simulink model "AcasXuClosedLoop_all"  (Simulink 10.0)
settings: Solver=VariableStepAuto  StartTime=0.0  StopTime=20  RelTol=1e-3
libraries: neural x14040, simulink x2
(part 36 of 534)

== system AcasXuClosedLoop_all/networks/Networks_m_9/AcasXu_1_9/Layer 3 ==
  "a{2} " : Inport  PortDimensions=50
      mask Display: e = 0.06;c1 = [120 230 180]/255;c2 = ([120 230 180]+0)/(256+128);c3 = ([120 230 180]+128)/(256+128);patch([0 1 1 0],[0 0 1 1],c1);patch([0 1 1 1-e 1-e 0+e],[0 0 1 1-e 0+e 0+e],c2);patch([0 0 1 1-e 0+e 0+e],[0 1 1 1-e 1-e 0+e],c3);plot([0 1 1 0 0],[0 0 1 1 0]);
  Delays 1 : Subsystem
      mask Display: e = 0.06;c1 = [120 150 230]/255;c2 = ([120 150 230]+0)/383;c3 = ([120 150 230]+128)/383;patch([0 1 1 0],[0 0 1 1],c1);patch([0 1 1 1-e 1-e 0+e],[0 0 1 1-e 0+e 0+e],c2);patch([0 0 1 1-e 0+e 0+e],[0 1 1 1-e 1-e 0+e],c3);plot([0 1 1 0 0],[0 0 1 1 0]);disp('0');
  "LW{3,2}" : Subsystem
      mask Display: e = 0.06;c1 = [120 150 230]/255;c2 = ([120 150 230]+0)/383;c3 = ([120 150 230]+128)/383;patch([0 1 1 0],[0 0 1 1],c1);patch([0 1 1 1-e 1-e 0+e],[0 0 1 1-e 0+e 0+e],c2);patch([0 0 1 1-e 0+e 0+e],[0 1 1 1-e 1-e 0+e],c3);plot([0 1 1 0 0],[0 0 1 1 0]);disp('W');
  "b{3}" : Constant  Value=[0.579304000000000041126213545794598758220672607421875;0.1281029999999999946513895565658458508551120758056640625;-0.1748010000000000119246834628938813693821430206298828125;-0.0029703199999999998180688631777002228773199021816253662109375;-0.057232600000000001527755699726185412146151065826416015625;-0.257135999999999975695885723325773142278194427490234375;-0.0175342999999999991922461362037211074493825435638427734375;0.2345440000000000024815705046421498991549015045166015625;-0.000781700999999999988736287814816705576959066092967987060546875;-0.00547454999999999990245580505643374635837972164154052734375;-0.04520269999999999843698361701171961612999439239501953125;-0.2583699999999999885602619542623870074748992919921875;-0.0831357000000000068151706500430009327828884124755859375;0.06992959999999999454178833957485039718449115753173828125;-0.04588620000000000198614458213342004455626010894775390625;-0.162262999999999990574650610142271034419536590576171875;0.000353693999999999991908083973868315297295339405536651611328125;0.2176039999999999918767201734226546250283718109130859375;0.1180549999999999932764893628700519911944866180419921875;0.044857800000000003226574563086614944040775299072265625;-0.6526009999999999866560074224253185093402862548828125;0.4370629999999999792947846799506805837154388427734375;0.06949550000000000171684888528034207411110401153564453125;-0.05631350000000000244337883259504451416432857513427734375;-0.2005689999999999972857267493964172899723052978515625;-0.61900800000000000267164068645797669887542724609375;0.07579249999999999876099110451832530088722705841064453125;0.164617999999999986560084153097704984247684478759765625;-0.6353269999999999750883716842508874833583831787109375;0.0186143000000000002902567075580009259283542633056640625;0.18131099999999999994315658113919198513031005859375;0.047283499999999999141575557359828962944447994232177734375;-0.340189000000000019152679442413500510156154632568359375;0.43907200000000001782751724022091366350650787353515625;0.263168000000000013027801060161436907947063446044921875;0.017979900000000000048760995241536875255405902862548828125;-0.00601458000000000027329694063382703461684286594390869140625;0.08392919999999999547402040889210184104740619659423828125;0.59921100000000004914824103252612985670566558837890625;0.10220300000000000217870166352440719492733478546142578125;0.303765000000000007229772336359019391238689422607421875;0.163652999999999992919441638150601647794246673583984375;-0.029870500000000001217248524199021630920469760894775390625;0.1906400000000000038991032624835497699677944183349609375;0.01548440000000000059399152263495125225745141506195068359375;-0.2505100000000000104449782156734727323055267333984375;-0.1780980000000000063042904230314888991415500640869140625;-0.2264130000000000031423752488990430720150470733642578125;-0.048898299999999998710631388121328200213611125946044921875;-0.12159599999999999575539533225310151465237140655517578125]
      mask Display: e = 0.06;c1 = [120 150 230]/255;c2 = ([120 150 230]+0)/383;c3 = ([120 150 230]+128)/383;patch([0 1 1 0],[0 0 1 1],c1);patch([0 1 1 1-e 1-e 0+e],[0 0 1 1-e 0+e 0+e],c2);patch([0 0 1 1-e 0+e 0+e],[0 1 1 1-e 1-e 0+e],c3);plot([0 1 1 0 0],[0 0 1 1 0]);disp('b');
  netsum : Sum  Inputs=2  SaturateOnIntegerOverflow=on  InputSameDT=on  OutDataTypeStr=Inherit: Same as first input
      mask Display: e = 0.06;c1 = [120 150 230]/255;c2 = ([120 150 230]+0)/383;c3 = ([120 150 230]+128)/383;patch([0 1 1 0],[0 0 1 1],c1);patch([0 1 1 1-e 1-e 0+e],[0 0 1 1-e 0+e 0+e],c2);patch([0 0 1 1-e 0+e 0+e],[0 1 1 1-e 1-e 0+e],c3);plot([0 1 1 0 0],[0 0 1 1 0]);disp('+');
  poslin : neural/Transfer Functions/poslin
      settings: SourceProductName=Deep Learning Toolbox  SourceProductBaseCode=NN
  "a{3}" : Outport  InitialOutput=[0;0;0;0;0;0;0;0;0;0;0;0;0;0;0;0;0;0;0;0;0;0;0;0;0;0;0;0;0;0;0;0;0;0;0;0;0;0;0;0;0;0;0;0;0;0;0;0;0;0]
      mask Display: e = 0.06;c1 = [120 230 180]/255;c2 = ([120 230 180]+0)/(256+128);c3 = ([120 230 180]+128)/(256+128);patch([0 1 1 0],[0 0 1 1],c1);patch([0 1 1 1-e 1-e 0+e],[0 0 1 1-e 0+e 0+e],c2);patch([0 0 1 1-e 0+e 0+e],[0 1 1 1-e 1-e 0+e],c3);plot([0 1 1 0 0],[0 0 1 1 0]);
  wires:
  poslin/1 -> "a{3}"/1
  netsum/1 -> poslin/1
  "b{3}"/1 -> netsum/2
  "LW{3,2}"/1("lz{3,2}") -> netsum/1
  Delays 1/1("pd{3,2}") -> "LW{3,2}"/1("ad{3,2}")
  "a{2} "/1 -> Delays 1/1("p{3}")

== system AcasXuClosedLoop_all/networks/Networks_m_9/AcasXu_1_9/Layer 4 ==
  "a{3} " : Inport  PortDimensions=50
      mask Display: e = 0.06;c1 = [120 230 180]/255;c2 = ([120 230 180]+0)/(256+128);c3 = ([120 230 180]+128)/(256+128);patch([0 1 1 0],[0 0 1 1],c1);patch([0 1 1 1-e 1-e 0+e],[0 0 1 1-e 0+e 0+e],c2);patch([0 0 1 1-e 0+e 0+e],[0 1 1 1-e 1-e 0+e],c3);plot([0 1 1 0 0],[0 0 1 1 0]);
  Delays 1 : Subsystem
      mask Display: e = 0.06;c1 = [120 150 230]/255;c2 = ([120 150 230]+0)/383;c3 = ([120 150 230]+128)/383;patch([0 1 1 0],[0 0 1 1],c1);patch([0 1 1 1-e 1-e 0+e],[0 0 1 1-e 0+e 0+e],c2);patch([0 0 1 1-e 0+e 0+e],[0 1 1 1-e 1-e 0+e],c3);plot([0 1 1 0 0],[0 0 1 1 0]);disp('0');
  "LW{4,3}" : Subsystem
      mask Display: e = 0.06;c1 = [120 150 230]/255;c2 = ([120 150 230]+0)/383;c3 = ([120 150 230]+128)/383;patch([0 1 1 0],[0 0 1 1],c1);patch([0 1 1 1-e 1-e 0+e],[0 0 1 1-e 0+e 0+e],c2);patch([0 0 1 1-e 0+e 0+e],[0 1 1 1-e 1-e 0+e],c3);plot([0 1 1 0 0],[0 0 1 1 0]);disp('W');
  "b{4}" : Constant  Value=[0.00952038000000000016187495788244632421992719173431396484375;-0.343938000000000021483259615706629119813442230224609375;1.177820000000000089102059064316563308238983154296875;-0.228142000000000011450396186774014495313167572021484375;-0.294987999999999972455810848259716294705867767333984375;-0.03578710000000000224229523837493616156280040740966796875;-0.257523000000000001907807245515868999063968658447265625;-0.255493000000000025639934619903215207159519195556640625;-0.280859000000000025298874106738367117941379547119140625;-0.2443720000000000058815174952542292885482311248779296875;-0.1862540000000000028901325777042075060307979583740234375;-0.08710220000000000462048177496399148367345333099365234375;-0.050596099999999998020339120330390869639813899993896484375;-0.04549129999999999840465392253463505767285823822021484375;-0.1145899999999999974154007986726355738937854766845703125;-0.1090150000000000007904787935331114567816257476806640625;-0.81820400000000004236966333337477408349514007568359375;-0.252141000000000004011013743365765549242496490478515625;0.43712099999999998178878968246863223612308502197265625;-0.0166662999999999984768184191352702328003942966461181640625;-0.317574000000000022936319510336033999919891357421875;-0.06494189999999999685087459511123597621917724609375;-0.8847620000000000484163820146932266652584075927734375;-0.02530469999999999941575623552125762216746807098388671875;0.07968530000000000057536198028174112550914287567138671875;-0.2003979999999999928039784435895853675901889801025390625;0.320222999999999979880982436952763237059116363525390625;-0.333703000000000027380764322515460662543773651123046875;0.1671620000000000050288662123421090655028820037841796875;-0.014145100000000000728395121996072703041136264801025390625;-0.37539800000000000945732381296693347394466400146484375;-0.90470600000000001017497197608463466167449951171875;0.05014369999999999938378181241205311380326747894287109375;-0.257811999999999985622167741894372738897800445556640625;-0.00607064000000000041079584178760342183522880077362060546875;0.34403600000000000846966941026039421558380126953125;-0.0242753999999999990622168155596227734349668025970458984375;-0.295009000000000021213253376117791049182415008544921875;-0.1626789999999999902779990179624292068183422088623046875;-0.325608999999999981778131541432230733335018157958984375;0.4586040000000000116386900117504410445690155029296875;0.15100700000000000233768560065072961151599884033203125;-0.01311299999999999965627495157605153508484363555908203125;-0.380357000000000000650146603220491670072078704833984375;0.0221107000000000004924505248027344350703060626983642578125;-0.10982599999999999307309650475872331298887729644775390625;-0.1503339999999999954116702838291530497372150421142578125;-0.01328509999999999934006122970231444924138486385345458984375;-0.04880589999999999928359528666987898759543895721435546875;-0.046639199999999998713651194748308626003563404083251953125]
      mask Display: e = 0.06;c1 = [120 150 230]/255;c2 = ([120 150 230]+0)/383;c3 = ([120 150 230]+128)/383;patch([0 1 1 0],[0 0 1 1],c1);patch([0 1 1 1-e 1-e 0+e],[0 0 1 1-e 0+e 0+e],c2);patch([0 0 1 1-e 0+e 0+e],[0 1 1 1-e 1-e 0+e],c3);plot([0 1 1 0 0],[0 0 1 1 0]);disp('b');
  netsum : Sum  Inputs=2  SaturateOnIntegerOverflow=on  InputSameDT=on  OutDataTypeStr=Inherit: Same as first input
      mask Display: e = 0.06;c1 = [120 150 230]/255;c2 = ([120 150 230]+0)/383;c3 = ([120 150 230]+128)/383;patch([0 1 1 0],[0 0 1 1],c1);patch([0 1 1 1-e 1-e 0+e],[0 0 1 1-e 0+e 0+e],c2);patch([0 0 1 1-e 0+e 0+e],[0 1 1 1-e 1-e 0+e],c3);plot([0 1 1 0 0],[0 0 1 1 0]);disp('+');
  poslin : neural/Transfer Functions/poslin
      settings: SourceProductName=Deep Learning Toolbox  SourceProductBaseCode=NN
  "a{4}" : Outport  InitialOutput=[0;0;0;0;0;0;0;0;0;0;0;0;0;0;0;0;0;0;0;0;0;0;0;0;0;0;0;0;0;0;0;0;0;0;0;0;0;0;0;0;0;0;0;0;0;0;0;0;0;0]
      mask Display: e = 0.06;c1 = [120 230 180]/255;c2 = ([120 230 180]+0)/(256+128);c3 = ([120 230 180]+128)/(256+128);patch([0 1 1 0],[0 0 1 1],c1);patch([0 1 1 1-e 1-e 0+e],[0 0 1 1-e 0+e 0+e],c2);patch([0 0 1 1-e 0+e 0+e],[0 1 1 1-e 1-e 0+e],c3);plot([0 1 1 0 0],[0 0 1 1 0]);
  wires:
  poslin/1 -> "a{4}"/1
  netsum/1 -> poslin/1
  "b{4}"/1 -> netsum/2
  "LW{4,3}"/1("lz{4,3}") -> netsum/1
  Delays 1/1("pd{4,3}") -> "LW{4,3}"/1("ad{4,3}")
  "a{3} "/1 -> Delays 1/1("p{4}")

== system AcasXuClosedLoop_all/networks/Networks_m_9/AcasXu_1_9/Layer 5 ==
  "a{4} " : Inport  PortDimensions=50
      mask Display: e = 0.06;c1 = [120 230 180]/255;c2 = ([120 230 180]+0)/(256+128);c3 = ([120 230 180]+128)/(256+128);patch([0 1 1 0],[0 0 1 1],c1);patch([0 1 1 1-e 1-e 0+e],[0 0 1 1-e 0+e 0+e],c2);patch([0 0 1 1-e 0+e 0+e],[0 1 1 1-e 1-e 0+e],c3);plot([0 1 1 0 0],[0 0 1 1 0]);
  Delays 1 : Subsystem
      mask Display: e = 0.06;c1 = [120 150 230]/255;c2 = ([120 150 230]+0)/383;c3 = ([120 150 230]+128)/383;patch([0 1 1 0],[0 0 1 1],c1);patch([0 1 1 1-e 1-e 0+e],[0 0 1 1-e 0+e 0+e],c2);patch([0 0 1 1-e 0+e 0+e],[0 1 1 1-e 1-e 0+e],c3);plot([0 1 1 0 0],[0 0 1 1 0]);disp('0');
  "LW{5,4}" : Subsystem
      mask Display: e = 0.06;c1 = [120 150 230]/255;c2 = ([120 150 230]+0)/383;c3 = ([120 150 230]+128)/383;patch([0 1 1 0],[0 0 1 1],c1);patch([0 1 1 1-e 1-e 0+e],[0 0 1 1-e 0+e 0+e],c2);patch([0 0 1 1-e 0+e 0+e],[0 1 1 1-e 1-e 0+e],c3);plot([0 1 1 0 0],[0 0 1 1 0]);disp('W');
  "b{5}" : Constant  Value=[-0.29072399999999998243538357201032340526580810546875;0.0272885999999999996623589737509973929263651371002197265625;0.1793730000000000046611603465862572193145751953125;-0.07173649999999999471445022436455474235117435455322265625;-0.234843999999999997196908907426404766738414764404296875;-0.85785699999999998066613215996767394244670867919921875;-0.00822291999999999988213428281369488104246556758880615234375;0.265643000000000018001600210482138209044933319091796875;-1.3297399999999999220534618871170096099376678466796875;0.1350659999999999916209247885490185581147670745849609375;-0.148589999999999999857891452847979962825775146484375;0.08024480000000000501358954352326691150665283203125;0.26909199999999999786126636536209844052791595458984375;-0.285260000000000013553602684623911045491695404052734375;0.1955969999999999930917482515724259428679943084716796875;0.3249020000000000241158204516978003084659576416015625;0.494925000000000003819167204710538499057292938232421875;-0.06360369999999999912621007069901679642498493194580078125;-0.014471299999999999441602227534531266428530216217041015625;-0.302879999999999982573939405483542941510677337646484375;-0.1371610000000000051390003363849245943129062652587890625;-1.131040000000000045332626541494391858577728271484375;-0.440622999999999986897591952583752572536468505859375;0.03643760000000000054409809990829671733081340789794921875;0.1914639999999999953050888734651380218565464019775390625;0.17777399999999998758681840627104975283145904541015625;-0.449446999999999985409004921166342683136463165283203125;-0.1299329999999999929460869907416054047644138336181640625;-0.018397299999999998376676302314081112854182720184326171875;-0.019312400000000000399591471023086342029273509979248046875;0.10747600000000000208810746471499442122876644134521484375;0.1691249999999999975575093458246556110680103302001953125;-0.044454500000000000958788604066285188309848308563232421875;0.250553999999999998937738610038650222122669219970703125;-0.331932999999999978069098460764507763087749481201171875;0.1414890000000000036539660186463152058422565460205078125;0.1969249999999999889421786747334408573806285858154296875;-0.768086999999999964217067827121354639530181884765625;0.06017229999999999801030270418777945451438426971435546875;-1.236050000000000093081098384573124349117279052734375;-0.93613400000000002165734258596785366535186767578125;-0.1641060000000000018705037518884637393057346343994140625;-0.217586000000000001630695578569429926574230194091796875;-0.0254946999999999986130649887172694434411823749542236328125;-0.174792000000000002923883357652812264859676361083984375;-0.1638570000000000026485480475457734428346157073974609375;-2.14449999999999985078602549037896096706390380859375;-0.459907000000000010242473535981844179332256317138671875;-0.09570120000000000015649703755116206593811511993408203125;0.132589000000000012402523452692548744380474090576171875]
      mask Display: e = 0.06;c1 = [120 150 230]/255;c2 = ([120 150 230]+0)/383;c3 = ([120 150 230]+128)/383;patch([0 1 1 0],[0 0 1 1],c1);patch([0 1 1 1-e 1-e 0+e],[0 0 1 1-e 0+e 0+e],c2);patch([0 0 1 1-e 0+e 0+e],[0 1 1 1-e 1-e 0+e],c3);plot([0 1 1 0 0],[0 0 1 1 0]);disp('b');
  netsum : Sum  Inputs=2  SaturateOnIntegerOverflow=on  InputSameDT=on  OutDataTypeStr=Inherit: Same as first input
      mask Display: e = 0.06;c1 = [120 150 230]/255;c2 = ([120 150 230]+0)/383;c3 = ([120 150 230]+128)/383;patch([0 1 1 0],[0 0 1 1],c1);patch([0 1 1 1-e 1-e 0+e],[0 0 1 1-e 0+e 0+e],c2);patch([0 0 1 1-e 0+e 0+e],[0 1 1 1-e 1-e 0+e],c3);plot([0 1 1 0 0],[0 0 1 1 0]);disp('+');
  poslin : neural/Transfer Functions/poslin
      settings: SourceProductName=Deep Learning Toolbox  SourceProductBaseCode=NN
  "a{5}" : Outport  InitialOutput=[0;0;0;0;0;0;0;0;0;0;0;0;0;0;0;0;0;0;0;0;0;0;0;0;0;0;0;0;0;0;0;0;0;0;0;0;0;0;0;0;0;0;0;0;0;0;0;0;0;0]
      mask Display: e = 0.06;c1 = [120 230 180]/255;c2 = ([120 230 180]+0)/(256+128);c3 = ([120 230 180]+128)/(256+128);patch([0 1 1 0],[0 0 1 1],c1);patch([0 1 1 1-e 1-e 0+e],[0 0 1 1-e 0+e 0+e],c2);patch([0 0 1 1-e 0+e 0+e],[0 1 1 1-e 1-e 0+e],c3);plot([0 1 1 0 0],[0 0 1 1 0]);
  wires:
  poslin/1 -> "a{5}"/1
  netsum/1 -> poslin/1
  "b{5}"/1 -> netsum/2
  "LW{5,4}"/1("lz{5,4}") -> netsum/1
  Delays 1/1("pd{5,4}") -> "LW{5,4}"/1("ad{5,4}")
  "a{4} "/1 -> Delays 1/1("p{5}")

== system AcasXuClosedLoop_all/networks/Networks_m_9/AcasXu_1_9/Layer 6 ==
  "a{5} " : Inport  PortDimensions=50
      mask Display: e = 0.06;c1 = [120 230 180]/255;c2 = ([120 230 180]+0)/(256+128);c3 = ([120 230 180]+128)/(256+128);patch([0 1 1 0],[0 0 1 1],c1);patch([0 1 1 1-e 1-e 0+e],[0 0 1 1-e 0+e 0+e],c2);patch([0 0 1 1-e 0+e 0+e],[0 1 1 1-e 1-e 0+e],c3);plot([0 1 1 0 0],[0 0 1 1 0]);
  Delays 1 : Subsystem
      mask Display: e = 0.06;c1 = [120 150 230]/255;c2 = ([120 150 230]+0)/383;c3 = ([120 150 230]+128)/383;patch([0 1 1 0],[0 0 1 1],c1);patch([0 1 1 1-e 1-e 0+e],[0 0 1 1-e 0+e 0+e],c2);patch([0 0 1 1-e 0+e 0+e],[0 1 1 1-e 1-e 0+e],c3);plot([0 1 1 0 0],[0 0 1 1 0]);disp('0');
  "LW{6,5}" : Subsystem
      mask Display: e = 0.06;c1 = [120 150 230]/255;c2 = ([120 150 230]+0)/383;c3 = ([120 150 230]+128)/383;patch([0 1 1 0],[0 0 1 1],c1);patch([0 1 1 1-e 1-e 0+e],[0 0 1 1-e 0+e 0+e],c2);patch([0 0 1 1-e 0+e 0+e],[0 1 1 1-e 1-e 0+e],c3);plot([0 1 1 0 0],[0 0 1 1 0]);disp('W');
  "b{6}" : Constant  Value=[0.054117800000000000626432239414498326368629932403564453125;-0.029863699999999999967759123364885454066097736358642578125;-0.3050450000000000105870867628254927694797515869140625;-0.04563230000000000063931082650015014223754405975341796875;-0.91212400000000004585132273859926499426364898681640625;-0.38360300000000002729194648054544813930988311767578125;-1.2402599999999999180744225668604485690593719482421875;0.03821739999999999859436883298258180730044841766357421875;-0.003371550000000000067712502271888297400437295436859130859375;0.7082969999999999544115780736319720745086669921875;-0.0424785000000000023678836669205338694155216217041015625;-0.463994999999999990780708003512700088322162628173828125;-0.048301900000000001778044378397680702619254589080810546875;-0.47921599999999997532285078705172054469585418701171875;0.07510529999999999983817389193063718266785144805908203125;-0.053192499999999996729282969454288831911981105804443359375;0.1580640000000000100488506404872168786823749542236328125;-0.06372360000000000523900922644315869547426700592041015625;0.007425969999999999619777479864524138974957168102264404296875;-0.237055999999999988947507745251641608774662017822265625;-0.7746969999999999689777041567140258848667144775390625;0.000659087000000000040877967677488413755781948566436767578125;-0.357331999999999982975396051187999546527862548828125;0.0168764999999999991409094235450538690201938152313232421875;-0.0990453000000000027824853532365523278713226318359375;0.09667389999999999317115140229361713863909244537353515625;-0.045709800000000001818367678652066388167440891265869140625;0.1134050000000000057998050806418177671730518341064453125;0.1596950000000000036148861681795096956193447113037109375;-0.122028999999999998582467242158600129187107086181640625;-0.29037400000000002098232698699575848877429962158203125;-0.0639891999999999960824226263866876251995563507080078125;-0.0760119999999999962358998573108692653477191925048828125;-0.07896699999999999552979801364926970563828945159912109375;-0.3772699999999999942446038403431884944438934326171875;-0.04114099999999999701660868822727934457361698150634765625;0.0158231999999999990713650532825340633280575275421142578125;-0.76031700000000002059863390968530438840389251708984375;-0.2510149999999999881339363128063268959522247314453125;0.0304802999999999983671283843023047666065394878387451171875;-0.09740379999999999871551636942967888899147510528564453125;-0.03860149999999999692601448941786657087504863739013671875;0.05038600000000000023181456754173268564045429229736328125;0.05410210000000000019060308886764687485992908477783203125;-0.035352899999999999602895428552074008621275424957275390625;-0.268838999999999994638955058690044097602367401123046875;-0.04333900000000000252153853352865553461015224456787109375;-0.026029400000000001147437700410591787658631801605224609375;-0.1915530000000000010462741784067475236952304840087890625;0.041969499999999999861888255736630526371300220489501953125]
      mask Display: e = 0.06;c1 = [120 150 230]/255;c2 = ([120 150 230]+0)/383;c3 = ([120 150 230]+128)/383;patch([0 1 1 0],[0 0 1 1],c1);patch([0 1 1 1-e 1-e 0+e],[0 0 1 1-e 0+e 0+e],c2);patch([0 0 1 1-e 0+e 0+e],[0 1 1 1-e 1-e 0+e],c3);plot([0 1 1 0 0],[0 0 1 1 0]);disp('b');
  netsum : Sum  Inputs=2  SaturateOnIntegerOverflow=on  InputSameDT=on  OutDataTypeStr=Inherit: Same as first input
      mask Display: e = 0.06;c1 = [120 150 230]/255;c2 = ([120 150 230]+0)/383;c3 = ([120 150 230]+128)/383;patch([0 1 1 0],[0 0 1 1],c1);patch([0 1 1 1-e 1-e 0+e],[0 0 1 1-e 0+e 0+e],c2);patch([0 0 1 1-e 0+e 0+e],[0 1 1 1-e 1-e 0+e],c3);plot([0 1 1 0 0],[0 0 1 1 0]);disp('+');
  poslin : neural/Transfer Functions/poslin
      settings: SourceProductName=Deep Learning Toolbox  SourceProductBaseCode=NN
  "a{6}" : Outport  InitialOutput=[0;0;0;0;0;0;0;0;0;0;0;0;0;0;0;0;0;0;0;0;0;0;0;0;0;0;0;0;0;0;0;0;0;0;0;0;0;0;0;0;0;0;0;0;0;0;0;0;0;0]
      mask Display: e = 0.06;c1 = [120 230 180]/255;c2 = ([120 230 180]+0)/(256+128);c3 = ([120 230 180]+128)/(256+128);patch([0 1 1 0],[0 0 1 1],c1);patch([0 1 1 1-e 1-e 0+e],[0 0 1 1-e 0+e 0+e],c2);patch([0 0 1 1-e 0+e 0+e],[0 1 1 1-e 1-e 0+e],c3);plot([0 1 1 0 0],[0 0 1 1 0]);
  wires:
  poslin/1 -> "a{6}"/1
  netsum/1 -> poslin/1
  "b{6}"/1 -> netsum/2
  "LW{6,5}"/1("lz{6,5}") -> netsum/1
  Delays 1/1("pd{6,5}") -> "LW{6,5}"/1("ad{6,5}")
  "a{5} "/1 -> Delays 1/1("p{6}")

== system AcasXuClosedLoop_all/networks/Networks_m_9/AcasXu_1_9/Layer 7 ==
  "a{6} " : Inport  PortDimensions=50
      mask Display: e = 0.06;c1 = [120 230 180]/255;c2 = ([120 230 180]+0)/(256+128);c3 = ([120 230 180]+128)/(256+128);patch([0 1 1 0],[0 0 1 1],c1);patch([0 1 1 1-e 1-e 0+e],[0 0 1 1-e 0+e 0+e],c2);patch([0 0 1 1-e 0+e 0+e],[0 1 1 1-e 1-e 0+e],c3);plot([0 1 1 0 0],[0 0 1 1 0]);
  Delays 1 : Subsystem
      mask Display: e = 0.06;c1 = [120 150 230]/255;c2 = ([120 150 230]+0)/383;c3 = ([120 150 230]+128)/383;patch([0 1 1 0],[0 0 1 1],c1);patch([0 1 1 1-e 1-e 0+e],[0 0 1 1-e 0+e 0+e],c2);patch([0 0 1 1-e 0+e 0+e],[0 1 1 1-e 1-e 0+e],c3);plot([0 1 1 0 0],[0 0 1 1 0]);disp('0');
  "LW{7,6}" : Subsystem
      mask Display: e = 0.06;c1 = [120 150 230]/255;c2 = ([120 150 230]+0)/383;c3 = ([120 150 230]+128)/383;patch([0 1 1 0],[0 0 1 1],c1);patch([0 1 1 1-e 1-e 0+e],[0 0 1 1-e 0+e 0+e],c2);patch([0 0 1 1-e 0+e 0+e],[0 1 1 1-e 1-e 0+e],c3);plot([0 1 1 0 0],[0 0 1 1 0]);disp('W');
  "b{7}" : Constant  Value=[-0.0201197000000000007779998867363246972672641277313232421875;-0.019449399999999998633892772659237380139529705047607421875;-0.0194817000000000010995204746677700313739478588104248046875;-0.0195344999999999997808419749389940989203751087188720703125;-0.01952500000000000068833827526759705506265163421630859375]
      mask Display: e = 0.06;c1 = [120 150 230]/255;c2 = ([120 150 230]+0)/383;c3 = ([120 150 230]+128)/383;patch([0 1 1 0],[0 0 1 1],c1);patch([0 1 1 1-e 1-e 0+e],[0 0 1 1-e 0+e 0+e],c2);patch([0 0 1 1-e 0+e 0+e],[0 1 1 1-e 1-e 0+e],c3);plot([0 1 1 0 0],[0 0 1 1 0]);disp('b');
  netsum : Sum  Inputs=2  SaturateOnIntegerOverflow=on  InputSameDT=on  OutDataTypeStr=Inherit: Same as first input
      mask Display: e = 0.06;c1 = [120 150 230]/255;c2 = ([120 150 230]+0)/383;c3 = ([120 150 230]+128)/383;patch([0 1 1 0],[0 0 1 1],c1);patch([0 1 1 1-e 1-e 0+e],[0 0 1 1-e 0+e 0+e],c2);patch([0 0 1 1-e 0+e 0+e],[0 1 1 1-e 1-e 0+e],c3);plot([0 1 1 0 0],[0 0 1 1 0]);disp('+');
  purelin : neural/Transfer Functions/purelin
      settings: SourceProductName=Deep Learning Toolbox
  "a{7}" : Outport  InitialOutput=[0;0;0;0;0]
      mask Display: e = 0.06;c1 = [120 230 180]/255;c2 = ([120 230 180]+0)/(256+128);c3 = ([120 230 180]+128)/(256+128);patch([0 1 1 0],[0 0 1 1],c1);patch([0 1 1 1-e 1-e 0+e],[0 0 1 1-e 0+e 0+e],c2);patch([0 0 1 1-e 0+e 0+e],[0 1 1 1-e 1-e 0+e],c3);plot([0 1 1 0 0],[0 0 1 1 0]);
  wires:
  purelin/1 -> "a{7}"/1
  netsum/1 -> purelin/1
  "b{7}"/1 -> netsum/2
  "LW{7,6}"/1("lz{7,6}") -> netsum/1
  Delays 1/1("pd{7,6}") -> "LW{7,6}"/1("ad{7,6}")
  "a{6} "/1 -> Delays 1/1("p{7}")

== system AcasXuClosedLoop_all/networks/Networks_m_9/AcasXu_1_9/Process Input 1 ==
  x : Inport  PortDimensions=5
      mask Display: e = 0.06;c1 = [120 230 180]/255;c2 = ([120 230 180]+0)/(256+128);c3 = ([120 230 180]+128)/(256+128);patch([0 1 1 0],[0 0 1 1],c1);patch([0 1 1 1-e 1-e 0+e],[0 0 1 1-e 0+e 0+e],c2);patch([0 0 1 1-e 0+e 0+e],[0 1 1 1-e 1-e 0+e],c3);plot([0 1 1 0 0],[0 0 1 1 0]);
  p : Outport  PortDimensions=5
      mask Display: e = 0.06;c1 = [120 230 180]/255;c2 = ([120 230 180]+0)/(256+128);c3 = ([120 230 180]+128)/(256+128);patch([0 1 1 0],[0 0 1 1],c1);patch([0 1 1 1-e 1-e 0+e],[0 0 1 1-e 0+e 0+e],c2);patch([0 0 1 1-e 0+e 0+e],[0 1 1 1-e 1-e 0+e],c3);plot([0 1 1 0 0],[0 0 1 1 0]);
  wires:
  x/1 -> p/1

== system AcasXuClosedLoop_all/networks/Networks_m_9/AcasXu_1_9/Process Output 1 ==
  a : Inport  PortDimensions=5
      mask Display: e = 0.06;c1 = [120 230 180]/255;c2 = ([120 230 180]+0)/(256+128);c3 = ([120 230 180]+128)/(256+128);patch([0 1 1 0],[0 0 1 1],c1);patch([0 1 1 1-e 1-e 0+e],[0 0 1 1-e 0+e 0+e],c2);patch([0 0 1 1-e 0+e 0+e],[0 1 1 1-e 1-e 0+e],c3);plot([0 1 1 0 0],[0 0 1 1 0]);
  y : Outport  PortDimensions=5
      mask Display: e = 0.06;c1 = [120 230 180]/255;c2 = ([120 230 180]+0)/(256+128);c3 = ([120 230 180]+128)/(256+128);patch([0 1 1 0],[0 0 1 1],c1);patch([0 1 1 1-e 1-e 0+e],[0 0 1 1-e 0+e 0+e],c2);patch([0 0 1 1-e 0+e 0+e],[0 1 1 1-e 1-e 0+e],c3);plot([0 1 1 0 0],[0 0 1 1 0]);
  wires:
  a/1 -> y/1

== system AcasXuClosedLoop_all/networks/Networks_m_9/AcasXu_2_9/Layer 1 ==
  "p{1}" : Inport  PortDimensions=5
      mask Display: e = 0.06;c1 = [120 230 180]/255;c2 = ([120 230 180]+0)/(256+128);c3 = ([120 230 180]+128)/(256+128);patch([0 1 1 0],[0 0 1 1],c1);patch([0 1 1 1-e 1-e 0+e],[0 0 1 1-e 0+e 0+e],c2);patch([0 0 1 1-e 0+e 0+e],[0 1 1 1-e 1-e 0+e],c3);plot([0 1 1 0 0],[0 0 1 1 0]);
  Delays 1 : Subsystem
      mask Display: e = 0.06;c1 = [120 150 230]/255;c2 = ([120 150 230]+0)/383;c3 = ([120 150 230]+128)/383;patch([0 1 1 0],[0 0 1 1],c1);patch([0 1 1 1-e 1-e 0+e],[0 0 1 1-e 0+e 0+e],c2);patch([0 0 1 1-e 0+e 0+e],[0 1 1 1-e 1-e 0+e],c3);plot([0 1 1 0 0],[0 0 1 1 0]);disp('0');
  "IW{1,1}" : Subsystem
      mask Display: e = 0.06;c1 = [120 150 230]/255;c2 = ([120 150 230]+0)/383;c3 = ([120 150 230]+128)/383;patch([0 1 1 0],[0 0 1 1],c1);patch([0 1 1 1-e 1-e 0+e],[0 0 1 1-e 0+e 0+e],c2);patch([0 0 1 1-e 0+e 0+e],[0 1 1 1-e 1-e 0+e],c3);plot([0 1 1 0 0],[0 0 1 1 0]);disp('W');
  "b{1}" : Constant  Value=[0.3133690000000000086544105215580202639102935791015625;-0.2295379999999999920401450026474776677787303924560546875;-0.68179599999999995763033666662522591650485992431640625;0.093745899999999993124077946049510501325130462646484375;-0.370910999999999990706101016257889568805694580078125;-0.08987399999999999555821972307967371307313442230224609375;0.241037000000000001254107928616576828062534332275390625;-0.0899728999999999945469397744091111235320568084716796875;0.01202310000000000010655920590352252474986016750335693359375;0.083605600000000002136602006430621258914470672607421875;-0.071525000000000005240252676230738870799541473388671875;0.1094549999999999967403851997005403973162174224853515625;0.211033999999999999364064251494710333645343780517578125;-0.201085000000000013731238368563936091959476470947265625;0.037052899999999999725019961260841228067874908447265625;-0.283455000000000012505552149377763271331787109375;0.1366680000000000116955334306112490594387054443359375;-0.63761199999999995657873341770027764141559600830078125;0.09052100000000000423749924038929748348891735076904296875;-0.017260299999999999254196580977804842405021190643310546875;-0.31535699999999999842970055397017858922481536865234375;0.08521869999999999445439158307635807432234287261962890625;-0.478976000000000012857270803579012863337993621826171875;-0.40773599999999998733102302139741368591785430908203125;-0.1191939999999999943991468853710102848708629608154296875;0.11499099999999999599520350557213532738387584686279296875;-0.463243000000000015869972003201837651431560516357421875;-0.348663000000000000699884594723698683083057403564453125;-0.351308000000000009155343150268890894949436187744140625;-0.26433600000000001539746108392137102782726287841796875;-0.2139709999999999945341500051654293201863765716552734375;-0.0735065000000000023927526626721373759210109710693359375;0.1385329999999999894821911539111169986426830291748046875;-0.497441999999999995285548948231735266745090484619140625;0.14723900000000000876099193192203529179096221923828125;0.1211699999999999999289457264239899814128875732421875;0.046926900000000000556799051310008508153259754180908203125;0.2262450000000000016608936448392341844737529754638671875;0.0255250999999999984846343892286313348449766635894775390625;0.043976099999999997136601592728766263462603092193603515625;0.038636600000000000110222941884785541333258152008056640625;-0.020880900000000000849009751391349709592759609222412109375;-0.3269130000000000091375795818748883903026580810546875;-0.2049249999999999960476060323344427160918712615966796875;-0.1191190000000000026592061885821749456226825714111328125;0.1084320000000000006057376822354854084551334381103515625;0.208442999999999989402255096138105727732181549072265625;-0.006081070000000000051743054285680045722983777523040771484375;-0.50589099999999997958610720161232165992259979248046875;0.578389000000000041978864828706718981266021728515625]
      mask Display: e = 0.06;c1 = [120 150 230]/255;c2 = ([120 150 230]+0)/383;c3 = ([120 150 230]+128)/383;patch([0 1 1 0],[0 0 1 1],c1);patch([0 1 1 1-e 1-e 0+e],[0 0 1 1-e 0+e 0+e],c2);patch([0 0 1 1-e 0+e 0+e],[0 1 1 1-e 1-e 0+e],c3);plot([0 1 1 0 0],[0 0 1 1 0]);disp('b');
  netsum : Sum  Inputs=2  SaturateOnIntegerOverflow=on  InputSameDT=on  OutDataTypeStr=Inherit: Same as first input
      mask Display: e = 0.06;c1 = [120 150 230]/255;c2 = ([120 150 230]+0)/383;c3 = ([120 150 230]+128)/383;patch([0 1 1 0],[0 0 1 1],c1);patch([0 1 1 1-e 1-e 0+e],[0 0 1 1-e 0+e 0+e],c2);patch([0 0 1 1-e 0+e 0+e],[0 1 1 1-e 1-e 0+e],c3);plot([0 1 1 0 0],[0 0 1 1 0]);disp('+');
  poslin : neural/Transfer Functions/poslin
      settings: SourceProductName=Deep Learning Toolbox  SourceProductBaseCode=NN
  "a{1}" : Outport  InitialOutput=[0;0;0;0;0;0;0;0;0;0;0;0;0;0;0;0;0;0;0;0;0;0;0;0;0;0;0;0;0;0;0;0;0;0;0;0;0;0;0;0;0;0;0;0;0;0;0;0;0;0]
      mask Display: e = 0.06;c1 = [120 230 180]/255;c2 = ([120 230 180]+0)/(256+128);c3 = ([120 230 180]+128)/(256+128);patch([0 1 1 0],[0 0 1 1],c1);patch([0 1 1 1-e 1-e 0+e],[0 0 1 1-e 0+e 0+e],c2);patch([0 0 1 1-e 0+e 0+e],[0 1 1 1-e 1-e 0+e],c3);plot([0 1 1 0 0],[0 0 1 1 0]);
  wires:
  poslin/1 -> "a{1}"/1
  netsum/1 -> poslin/1
  "b{1}"/1 -> netsum/2
  "IW{1,1}"/1("iz{1,1}") -> netsum/1
  Delays 1/1("pd{1,1}") -> "IW{1,1}"/1("pd{1,1}")
  "p{1}"/1 -> Delays 1/1("p{1}")

== system AcasXuClosedLoop_all/networks/Networks_m_9/AcasXu_2_9/Layer 2 ==
  "a{1} " : Inport  PortDimensions=50
      mask Display: e = 0.06;c1 = [120 230 180]/255;c2 = ([120 230 180]+0)/(256+128);c3 = ([120 230 180]+128)/(256+128);patch([0 1 1 0],[0 0 1 1],c1);patch([0 1 1 1-e 1-e 0+e],[0 0 1 1-e 0+e 0+e],c2);patch([0 0 1 1-e 0+e 0+e],[0 1 1 1-e 1-e 0+e],c3);plot([0 1 1 0 0],[0 0 1 1 0]);
  Delays 1 : Subsystem
      mask Display: e = 0.06;c1 = [120 150 230]/255;c2 = ([120 150 230]+0)/383;c3 = ([120 150 230]+128)/383;patch([0 1 1 0],[0 0 1 1],c1);patch([0 1 1 1-e 1-e 0+e],[0 0 1 1-e 0+e 0+e],c2);patch([0 0 1 1-e 0+e 0+e],[0 1 1 1-e 1-e 0+e],c3);plot([0 1 1 0 0],[0 0 1 1 0]);disp('0');
  "LW{2,1}" : Subsystem
      mask Display: e = 0.06;c1 = [120 150 230]/255;c2 = ([120 150 230]+0)/383;c3 = ([120 150 230]+128)/383;patch([0 1 1 0],[0 0 1 1],c1);patch([0 1 1 1-e 1-e 0+e],[0 0 1 1-e 0+e 0+e],c2);patch([0 0 1 1-e 0+e 0+e],[0 1 1 1-e 1-e 0+e],c3);plot([0 1 1 0 0],[0 0 1 1 0]);disp('W');
  "b{2}" : Constant  Value=[0.3204409999999999758557578388717956840991973876953125;-0.00630298999999999963461672081166398129425942897796630859375;-1.173140000000000071622707764618098735809326171875;-0.01077690000000000071500583231909331516362726688385009765625;-0.00983794999999999981998843878727711853571236133575439453125;0.256141000000000007563727422166266478598117828369140625;-0.055605700000000000959854418169925338588654994964599609375;-0.99877400000000005064748620497994124889373779296875;0.04399809999999999832187569381858338601887226104736328125;-2.3228699999999999903366187936626374721527099609375;-0.48113499999999997935873352616908960044384002685546875;-1.24988000000000010203393685515038669109344482421875;0.85943000000000002724931391639984212815761566162109375;-0.00695779000000000015513368367692237370647490024566650390625;-0.034944999999999996898036869197312626056373119354248046875;0.58568900000000001515587655376293696463108062744140625;-0.93983200000000000073896444519050419330596923828125;0.8823659999999999836717279322328977286815643310546875;0.1936070000000000013162804179955855943262577056884765625;-0.0227493000000000000770938868299708701670169830322265625;-0.006574370000000000387185838945924842846579849720001220703125;-0.0197879000000000006276312802810934954322874546051025390625;0.88072600000000000886046791492844931781291961669921875;-0.316321999999999992070343068917281925678253173828125;0.07317099999999999992983390484369010664522647857666015625;-0.01792269999999999974260589397090370766818523406982421875;-0.0213239999999999992053023589733129483647644519805908203125;-0.24118600000000001148947603724082000553607940673828125;-0.02980850000000000166178182325893430970609188079833984375;-0.0062708599999999996288391201915146666578948497772216796875;-0.186483000000000009865885886028991080820560455322265625;-0.01896509999999999862563271335602621547877788543701171875;0.3171829999999999927240423858165740966796875;-0.477076000000000000067501559897209517657756805419921875;-0.01143599999999999998478994456263535539619624614715576171875;-0.318616999999999983561593808190082199871540069580078125;0.201365999999999989444887660283711738884449005126953125;-0.00842177000000000029744651186547343968413770198822021484375;-1.5037700000000000510880227011512033641338348388671875;-0.01182579999999999915971660158220402081497013568878173828125;1.6199600000000000665778543407213874161243438720703125;-1.15589999999999992752464095246978104114532470703125;0.329060999999999992393640013688127510249614715576171875;-0.245472999999999996756372411255142651498317718505859375;-0.1748139999999999971702635548354010097682476043701171875;2.336559999999999970299313645227812230587005615234375;0.94333900000000003860378683384624309837818145751953125;-0.1901509999999999867004163434103247709572315216064453125;0.7491849999999999898392388786305673420429229736328125;1.364500000000000046185277824406512081623077392578125]
      mask Display: e = 0.06;c1 = [120 150 230]/255;c2 = ([120 150 230]+0)/383;c3 = ([120 150 230]+128)/383;patch([0 1 1 0],[0 0 1 1],c1);patch([0 1 1 1-e 1-e 0+e],[0 0 1 1-e 0+e 0+e],c2);patch([0 0 1 1-e 0+e 0+e],[0 1 1 1-e 1-e 0+e],c3);plot([0 1 1 0 0],[0 0 1 1 0]);disp('b');
  netsum : Sum  Inputs=2  SaturateOnIntegerOverflow=on  InputSameDT=on  OutDataTypeStr=Inherit: Same as first input
      mask Display: e = 0.06;c1 = [120 150 230]/255;c2 = ([120 150 230]+0)/383;c3 = ([120 150 230]+128)/383;patch([0 1 1 0],[0 0 1 1],c1);patch([0 1 1 1-e 1-e 0+e],[0 0 1 1-e 0+e 0+e],c2);patch([0 0 1 1-e 0+e 0+e],[0 1 1 1-e 1-e 0+e],c3);plot([0 1 1 0 0],[0 0 1 1 0]);disp('+');
  poslin : neural/Transfer Functions/poslin
      settings: SourceProductName=Deep Learning Toolbox  SourceProductBaseCode=NN
  "a{2}" : Outport  InitialOutput=[0;0;0;0;0;0;0;0;0;0;0;0;0;0;0;0;0;0;0;0;0;0;0;0;0;0;0;0;0;0;0;0;0;0;0;0;0;0;0;0;0;0;0;0;0;0;0;0;0;0]
      mask Display: e = 0.06;c1 = [120 230 180]/255;c2 = ([120 230 180]+0)/(256+128);c3 = ([120 230 180]+128)/(256+128);patch([0 1 1 0],[0 0 1 1],c1);patch([0 1 1 1-e 1-e 0+e],[0 0 1 1-e 0+e 0+e],c2);patch([0 0 1 1-e 0+e 0+e],[0 1 1 1-e 1-e 0+e],c3);plot([0 1 1 0 0],[0 0 1 1 0]);
  wires:
  poslin/1 -> "a{2}"/1
  netsum/1 -> poslin/1
  "b{2}"/1 -> netsum/2
  "LW{2,1}"/1("lz{2,1}") -> netsum/1
  Delays 1/1("pd{2,1}") -> "LW{2,1}"/1("ad{2,1}")
  "a{1} "/1 -> Delays 1/1("p{2}")

== system AcasXuClosedLoop_all/networks/Networks_m_9/AcasXu_2_9/Layer 3 ==
  "a{2} " : Inport  PortDimensions=50
      mask Display: e = 0.06;c1 = [120 230 180]/255;c2 = ([120 230 180]+0)/(256+128);c3 = ([120 230 180]+128)/(256+128);patch([0 1 1 0],[0 0 1 1],c1);patch([0 1 1 1-e 1-e 0+e],[0 0 1 1-e 0+e 0+e],c2);patch([0 0 1 1-e 0+e 0+e],[0 1 1 1-e 1-e 0+e],c3);plot([0 1 1 0 0],[0 0 1 1 0]);
  Delays 1 : Subsystem
      mask Display: e = 0.06;c1 = [120 150 230]/255;c2 = ([120 150 230]+0)/383;c3 = ([120 150 230]+128)/383;patch([0 1 1 0],[0 0 1 1],c1);patch([0 1 1 1-e 1-e 0+e],[0 0 1 1-e 0+e 0+e],c2);patch([0 0 1 1-e 0+e 0+e],[0 1 1 1-e 1-e 0+e],c3);plot([0 1 1 0 0],[0 0 1 1 0]);disp('0');
  "LW{3,2}" : Subsystem
      mask Display: e = 0.06;c1 = [120 150 230]/255;c2 = ([120 150 230]+0)/383;c3 = ([120 150 230]+128)/383;patch([0 1 1 0],[0 0 1 1],c1);patch([0 1 1 1-e 1-e 0+e],[0 0 1 1-e 0+e 0+e],c2);patch([0 0 1 1-e 0+e 0+e],[0 1 1 1-e 1-e 0+e],c3);plot([0 1 1 0 0],[0 0 1 1 0]);disp('W');
  "b{3}" : Constant  Value=[-1.8993500000000000937916411203332245349884033203125;0.8458200000000000162714286489062942564487457275390625;-1.0242299999999999737809730504523031413555145263671875;0.0908319999999999960760277417648467235267162322998046875;-1.5125599999999999045741105874185450375080108642578125;-3.19686000000000003495870259939692914485931396484375;-2.978800000000000114397380457376129925251007080078125;0.07137970000000000425632862288694013841450214385986328125;-0.031126199999999999701305597454847884364426136016845703125;-0.7462379999999999569126885035075247287750244140625;1.4888600000000000722621962268021889030933380126953125;0.407391000000000003122835323665640316903591156005859375;-0.55142199999999996773425436913385055959224700927734375;0.161221000000000003193889597241650335490703582763671875;0.2294299999999999950528462022703024558722972869873046875;-1.3071200000000000596145355302724055945873260498046875;-0.23964499999999999690913909944356419146060943603515625;-1.5535900000000000265032440438517369329929351806640625;-0.45615499999999997715605104531277902424335479736328125;-0.456284999999999996145305658501456491649150848388671875;-0.0488682000000000005268674385661142878234386444091796875;-1.9298699999999999743494072390603832900524139404296875;1.09536999999999995480948200565762817859649658203125;0.1771540000000000059099392046846332959830760955810546875;-2.115180000000000060111915445304475724697113037109375;-0.9154489999999999572111164525267668068408966064453125;-0.15679100000000001369215851809713058173656463623046875;-0.021237999999999999933830707732340670190751552581787109375;-1.1092800000000000437694325228221714496612548828125;-1.3860900000000000442668124378542415797710418701171875;-1.423270000000000035100811146548949182033538818359375;-1.0635399999999999298694319804781116545200347900390625;-0.0239374000000000010379697101825513527728617191314697265625;1.2449399999999999355537738665589131414890289306640625;-0.026943399999999999294697516916130552999675273895263671875;-0.304115999999999997438493437584838829934597015380859375;-1.5718199999999999949551465761032886803150177001953125;-0.96798799999999995957722376260790042579174041748046875;-0.3200410000000000199094074559980072081089019775390625;-1.0801600000000000090238927441532723605632781982421875;-0.1613650000000000084288132029541884548962116241455078125;-1.0039700000000000290611978925880976021289825439453125;-0.77348499999999997811528373858891427516937255859375;-0.003944040000000000219249063349025163915939629077911376953125;0.72809800000000002295763579240883700549602508544921875;-0.76578599999999996672528368435450829565525054931640625;-0.66063199999999999700861508244997821748256683349609375;-1.208159999999999900666125540737994015216827392578125;-0.17723400000000000265032440438517369329929351806640625;-2.990200000000000191135995919466949999332427978515625]
      mask Display: e = 0.06;c1 = [120 150 230]/255;c2 = ([120 150 230]+0)/383;c3 = ([120 150 230]+128)/383;patch([0 1 1 0],[0 0 1 1],c1);patch([0 1 1 1-e 1-e 0+e],[0 0 1 1-e 0+e 0+e],c2);patch([0 0 1 1-e 0+e 0+e],[0 1 1 1-e 1-e 0+e],c3);plot([0 1 1 0 0],[0 0 1 1 0]);disp('b');
  netsum : Sum  Inputs=2  SaturateOnIntegerOverflow=on  InputSameDT=on  OutDataTypeStr=Inherit: Same as first input
      mask Display: e = 0.06;c1 = [120 150 230]/255;c2 = ([120 150 230]+0)/383;c3 = ([120 150 230]+128)/383;patch([0 1 1 0],[0 0 1 1],c1);patch([0 1 1 1-e 1-e 0+e],[0 0 1 1-e 0+e 0+e],c2);patch([0 0 1 1-e 0+e 0+e],[0 1 1 1-e 1-e 0+e],c3);plot([0 1 1 0 0],[0 0 1 1 0]);disp('+');
  poslin : neural/Transfer Functions/poslin
      settings: SourceProductName=Deep Learning Toolbox  SourceProductBaseCode=NN
  "a{3}" : Outport  InitialOutput=[0;0;0;0;0;0;0;0;0;0;0;0;0;0;0;0;0;0;0;0;0;0;0;0;0;0;0;0;0;0;0;0;0;0;0;0;0;0;0;0;0;0;0;0;0;0;0;0;0;0]
      mask Display: e = 0.06;c1 = [120 230 180]/255;c2 = ([120 230 180]+0)/(256+128);c3 = ([120 230 180]+128)/(256+128);patch([0 1 1 0],[0 0 1 1],c1);patch([0 1 1 1-e 1-e 0+e],[0 0 1 1-e 0+e 0+e],c2);patch([0 0 1 1-e 0+e 0+e],[0 1 1 1-e 1-e 0+e],c3);plot([0 1 1 0 0],[0 0 1 1 0]);
  wires:
  poslin/1 -> "a{3}"/1
  netsum/1 -> poslin/1
  "b{3}"/1 -> netsum/2
  "LW{3,2}"/1("lz{3,2}") -> netsum/1
  Delays 1/1("pd{3,2}") -> "LW{3,2}"/1("ad{3,2}")
  "a{2} "/1 -> Delays 1/1("p{3}")

== system AcasXuClosedLoop_all/networks/Networks_m_9/AcasXu_2_9/Layer 4 ==
  "a{3} " : Inport  PortDimensions=50
      mask Display: e = 0.06;c1 = [120 230 180]/255;c2 = ([120 230 180]+0)/(256+128);c3 = ([120 230 180]+128)/(256+128);patch([0 1 1 0],[0 0 1 1],c1);patch([0 1 1 1-e 1-e 0+e],[0 0 1 1-e 0+e 0+e],c2);patch([0 0 1 1-e 0+e 0+e],[0 1 1 1-e 1-e 0+e],c3);plot([0 1 1 0 0],[0 0 1 1 0]);
  Delays 1 : Subsystem
      mask Display: e = 0.06;c1 = [120 150 230]/255;c2 = ([120 150 230]+0)/383;c3 = ([120 150 230]+128)/383;patch([0 1 1 0],[0 0 1 1],c1);patch([0 1 1 1-e 1-e 0+e],[0 0 1 1-e 0+e 0+e],c2);patch([0 0 1 1-e 0+e 0+e],[0 1 1 1-e 1-e 0+e],c3);plot([0 1 1 0 0],[0 0 1 1 0]);disp('0');
  "LW{4,3}" : Subsystem
      mask Display: e = 0.06;c1 = [120 150 230]/255;c2 = ([120 150 230]+0)/383;c3 = ([120 150 230]+128)/383;patch([0 1 1 0],[0 0 1 1],c1);patch([0 1 1 1-e 1-e 0+e],[0 0 1 1-e 0+e 0+e],c2);patch([0 0 1 1-e 0+e 0+e],[0 1 1 1-e 1-e 0+e],c3);plot([0 1 1 0 0],[0 0 1 1 0]);disp('W');
  "b{4}" : Constant  Value=[-4.7583999999999999630517777404747903347015380859375;0.3795830000000000037374547900981269776821136474609375;-0.50699799999999994870591990547836758196353912353515625;1.090850000000000097344354799133725464344024658203125;-1.4955700000000000660094201521133072674274444580078125;1.214329999999999909476855464163236320018768310546875;0.491422999999999998710364934595418162643909454345703125;1.954849999999999976552089719916693866252899169921875;-0.377244999999999996997956941413576714694499969482421875;-1.7761400000000000520827825312153436243534088134765625;-1.0422800000000000952837808654294349253177642822265625;2.0903200000000001779199010343290865421295166015625;-4.3212799999999997879740476491861045360565185546875;1.022790000000000087965190687100403010845184326171875;-0.006889909999999999958009144762627329328097403049468994140625;0.2351830000000000031601388172930455766618251800537109375;1.331609999999999960351715344586409628391265869140625;-2.927160000000000206199501917581073939800262451171875;0.76379600000000003046096708203549496829509735107421875;-0.355895999999999990137666827649809420108795166015625;-3.29070000000000018047785488306544721126556396484375;-0.024015399999999999247624060672023915685713291168212890625;-6.02374999999999971578290569595992565155029296875;-0.428636999999999990240695524335023947060108184814453125;-0.4152109999999999967457142702187411487102508544921875;0.6870249999999999968025576890795491635799407958984375;0.1364430000000000087201357246158295311033725738525390625;1.148849999999999926814098216709680855274200439453125;0.99999400000000004951061782776378095149993896484375;1.457359999999999988773424774990417063236236572265625;1.2686800000000000299138491755002178251743316650390625;0.75088100000000002065547732854611240327358245849609375;-0.0265271000000000013729906100934385904110968112945556640625;-1.118230000000000057269744502264074981212615966796875;2.66772000000000009123368727159686386585235595703125;1.6641600000000000836308799989637918770313262939453125;-1.1721600000000000907363073565647937357425689697265625;1.2632499999999999840127884453977458178997039794921875;-1.730069999999999996731503415503539144992828369140625;-2.447060000000000012931877790833823382854461669921875;-2.04990000000000005542233338928781449794769287109375;-0.60530399999999995319654999548220075666904449462890625;-0.67252100000000003543476623235619626939296722412109375;-0.1620520000000000016004975122996256686747074127197265625;-0.407907999999999992812860227786586619913578033447265625;0.58067699999999999871391764827421866357326507568359375;-1.0716099999999999514699311475851573050022125244140625;-0.263624000000000024979129875646322034299373626708984375;-0.9426390000000000046753712013014592230319976806640625;-1.28052000000000010260237104375846683979034423828125]
      mask Display: e = 0.06;c1 = [120 150 230]/255;c2 = ([120 150 230]+0)/383;c3 = ([120 150 230]+128)/383;patch([0 1 1 0],[0 0 1 1],c1);patch([0 1 1 1-e 1-e 0+e],[0 0 1 1-e 0+e 0+e],c2);patch([0 0 1 1-e 0+e 0+e],[0 1 1 1-e 1-e 0+e],c3);plot([0 1 1 0 0],[0 0 1 1 0]);disp('b');
  netsum : Sum  Inputs=2  SaturateOnIntegerOverflow=on  InputSameDT=on  OutDataTypeStr=Inherit: Same as first input
      mask Display: e = 0.06;c1 = [120 150 230]/255;c2 = ([120 150 230]+0)/383;c3 = ([120 150 230]+128)/383;patch([0 1 1 0],[0 0 1 1],c1);patch([0 1 1 1-e 1-e 0+e],[0 0 1 1-e 0+e 0+e],c2);patch([0 0 1 1-e 0+e 0+e],[0 1 1 1-e 1-e 0+e],c3);plot([0 1 1 0 0],[0 0 1 1 0]);disp('+');
  poslin : neural/Transfer Functions/poslin
      settings: SourceProductName=Deep Learning Toolbox  SourceProductBaseCode=NN
  "a{4}" : Outport  InitialOutput=[0;0;0;0;0;0;0;0;0;0;0;0;0;0;0;0;0;0;0;0;0;0;0;0;0;0;0;0;0;0;0;0;0;0;0;0;0;0;0;0;0;0;0;0;0;0;0;0;0;0]
      mask Display: e = 0.06;c1 = [120 230 180]/255;c2 = ([120 230 180]+0)/(256+128);c3 = ([120 230 180]+128)/(256+128);patch([0 1 1 0],[0 0 1 1],c1);patch([0 1 1 1-e 1-e 0+e],[0 0 1 1-e 0+e 0+e],c2);patch([0 0 1 1-e 0+e 0+e],[0 1 1 1-e 1-e 0+e],c3);plot([0 1 1 0 0],[0 0 1 1 0]);
  wires:
  poslin/1 -> "a{4}"/1
  netsum/1 -> poslin/1
  "b{4}"/1 -> netsum/2
  "LW{4,3}"/1("lz{4,3}") -> netsum/1
  Delays 1/1("pd{4,3}") -> "LW{4,3}"/1("ad{4,3}")
  "a{3} "/1 -> Delays 1/1("p{4}")
